simulink model "benchmark"  (Simulink 7.9) (continued)
== system benchmark/Wind Field == (continued)
      | image(imread('anemometer.jpg'))
      | 
      | port_label('output',1,'v_rot [m/s]')
      | port_label('output',2,'v_nac [m/s]')
      | 
      | 
      | port_label('input',1,'U_x [m/s]')
      | port_label('input',2,'WC [m]')
      | port_label('input',3,'a')
  Wind Speed6 : Subsystem  mask(Wind){}
      mask help: Calculates average over rotor and nacelle wind speeds based on wake effects
      mask init:
      | p=get_param(gcb,'UserData');
      mask Display:
      | image(imread('anemometer.jpg'))
      | 
      | port_label('output',1,'v_rot [m/s]')
      | port_label('output',2,'v_nac [m/s]')
      | 
      | 
      | port_label('input',1,'U_x [m/s]')
      | port_label('input',2,'WC [m]')
      | port_label('input',3,'a')
  Wind Speed7 : Subsystem  mask(Wind){}
      mask help: Calculates average over rotor and nacelle wind speeds based on wake effects
      mask init:
      | p=get_param(gcb,'UserData');
      mask Display:
      | image(imread('anemometer.jpg'))
      | 
      | port_label('output',1,'v_rot [m/s]')
      | port_label('output',2,'v_nac [m/s]')
      | 
      | 
      | port_label('input',1,'U_x [m/s]')
      | port_label('input',2,'WC [m]')
      | port_label('input',3,'a')
  Wind Speed8 : Subsystem  mask(Wind){}
      mask help: Calculates average over rotor and nacelle wind speeds based on wake effects
      mask init:
      | p=get_param(gcb,'UserData');
      mask Display:
      | image(imread('anemometer.jpg'))
      | 
      | port_label('output',1,'v_rot [m/s]')
      | port_label('output',2,'v_nac [m/s]')
      | 
      | 
      | port_label('input',1,'U_x [m/s]')
      | port_label('input',2,'WC [m]')
      | port_label('input',3,'a')
  Wind Speed9 : Subsystem  mask(Wind){}
      mask help: Calculates average over rotor and nacelle wind speeds based on wake effects
      mask init:
      | p=get_param(gcb,'UserData');
      mask Display:
      | image(imread('anemometer.jpg'))
      | 
      | port_label('output',1,'v_rot [m/s]')
      | port_label('output',2,'v_nac [m/s]')
      | 
      | 
      | port_label('input',1,'U_x [m/s]')
      | port_label('input',2,'WC [m]')
      | port_label('input',3,'a')
  aconcat : Concatenate  NumInputs=10  Mode=Multidimensional array  ConcatenateDimension=2
  rate1 : RateTransition  Deterministic=off  OutPortSampleTime=SWFTTs
  rate2 : RateTransition  Deterministic=off  OutPortSampleTime=SWFTTs
  wcconcat : Concatenate  NumInputs=10  Mode=Multidimensional array  ConcatenateDimension=2
  V_nac [m/s] : Outport
  V_rot [m/s] : Outport  Port=2
  wires:
  rate2/1 -> V_nac [m/s]/1
  rate1/1 -> V_rot [m/s]/1
  CTrate/1 -> CTdemux/1
  Vnacmux/1 -> rate2/1
  Vrotmux/1 -> rate1/1
  CT/1 -> CTrate/1
  CTdemux/1 -> Wake1/2(CT)
  Ambient Field/2(Uy) -> Wake1/1(uy), Wake2/1(uy), Wake3/1(uy), Wake4/1(uy), Wake5/1(uy), Wake6/1(uy), Wake7/1(uy), Wake8/1(uy), Wake9/1(uy), Wake10/1(uy)
  aconcat/1 -> Wind Speed1/3(a), Wind Speed2/3(a), Wind Speed3/3(a), Wind Speed4/3(a), Wind Speed5/3(a), Wind Speed6/3(a), Wind Speed7/3(a), Wind Speed8/3(a), Wind Speed9/3(a), Wind Speed10/3(a)  "wakeDeficit"
  wcconcat/1 -> Wind Speed1/2(w_c), Wind Speed2/2(w_c), Wind Speed3/2(w_c), Wind Speed4/2(w_c), Wind Speed5/2(w_c), Wind Speed6/2(w_c), Wind Speed7/2(w_c), Wind Speed8/2(w_c), Wind Speed9/2(w_c), Wind Speed10/2(w_c)  "wakeCenter"
  Ambient Field/1(Ux) -> Wind Speed1/1(ux), Wind Speed2/1(ux), Wind Speed3/1(ux), Wind Speed4/1(ux), Wind Speed5/1(ux), Wind Speed6/1(ux), Wind Speed7/1(ux), Wind Speed8/1(ux), Wind Speed9/1(ux), Wind Speed10/1(ux)
  Wake1/1(w_c) -> wcconcat/1
  Wake1/2(a) -> aconcat/1
  Wind Speed1/1(v_rot) -> Vrotmux/1
  Wind Speed1/2(v_nac) -> Vnacmux/1
  CTdemux/2 -> Wake2/2(CT)
  Wake2/1(w_c) -> wcconcat/2
  Wake2/2(a) -> aconcat/2
  Wind Speed2/1(v_rot) -> Vrotmux/2
  Wind Speed2/2(v_nac) -> Vnacmux/2
  CTdemux/3 -> Wake3/2(CT)
  Wake3/1(w_c) -> wcconcat/3
  Wake3/2(a) -> aconcat/3
  Wind Speed3/1(v_rot) -> Vrotmux/3
  Wind Speed3/2(v_nac) -> Vnacmux/3
  CTdemux/4 -> Wake4/2(CT)
  Wake4/1(w_c) -> wcconcat/4
  Wake4/2(a) -> aconcat/4
  Wind Speed4/1(v_rot) -> Vrotmux/4
  Wind Speed4/2(v_nac) -> Vnacmux/4
  CTdemux/5 -> Wake5/2(CT)
  Wake5/1(w_c) -> wcconcat/5
  Wake5/2(a) -> aconcat/5
  Wind Speed5/1(v_rot) -> Vrotmux/5
  Wind Speed5/2(v_nac) -> Vnacmux/5
  CTdemux/6 -> Wake6/2(CT)
  Wake6/1(w_c) -> wcconcat/6
  Wake6/2(a) -> aconcat/6
  Wind Speed6/1(v_rot) -> Vrotmux/6
  Wind Speed6/2(v_nac) -> Vnacmux/6
  CTdemux/7 -> Wake7/2(CT)
  Wake7/1(w_c) -> wcconcat/7
  Wake7/2(a) -> aconcat/7
  Wind Speed7/1(v_rot) -> Vrotmux/7
  Wind Speed7/2(v_nac) -> Vnacmux/7
  CTdemux/8 -> Wake8/2(CT)
  Wake8/1(w_c) -> wcconcat/8
  Wake8/2(a) -> aconcat/8
  Wind Speed8/1(v_rot) -> Vrotmux/8
  Wind Speed8/2(v_nac) -> Vnacmux/8
  CTdemux/9 -> Wake9/2(CT)
  Wake9/1(w_c) -> wcconcat/9
  Wake9/2(a) -> aconcat/9
  Wind Speed9/1(v_rot) -> Vrotmux/9
  Wind Speed9/2(v_nac) -> Vnacmux/9
  CTdemux/10 -> Wake10/2(CT)
  Wake10/1(w_c) -> wcconcat/10
  Wake10/2(a) -> aconcat/10
  Wind Speed10/1(v_rot) -> Vrotmux/10
  Wind Speed10/2(v_nac) -> Vnacmux/10

== system benchmark/Network Load/Power Load ==
  Demux : Demux  Tag=STV Demux  Outputs=1
  FromWs : FromWorkspace  Tag=STV FromWs  VariableName=tuvar  SampleTime=0  SigBuilderData=DataTag1  VnvData=DataTag2
      SigBuilderData=|
      | common.afterFinalStr = 'Extrapolate'
      | common.sampleTime = 0
      | dataSet.timeRange = [0 1000]
      | dataSet.name = 'Group 1'
      | sbobj.Groups.Name = 'Group 1'
      | sbobj.Groups.Signals.Name = 'Signal 1'
      | sbobj.Groups.Signals.XData = [0 25 100 200 500 1000]
      | sbobj.Groups.Signals.YData = [0.1 0.1 0.7 0.7 0.9 0.85]
  Signal 1 : Outport  Tag=STV Outport
  wires:
  FromWs/1 -> Demux/1
  Demux/1 -> Signal 1/1

== system benchmark/Post processing/Fatigue Computation ==

== system benchmark/Post processing/Wake Animation ==

== system benchmark/Post processing/Wind Field Animation ==

== system benchmark/Wind Field/Ambient Field ==
  Counter Free-Running : simulink/Sources/Counter Free-Running  NumBits=32  tsamp=wind.Ts
  Selector : Selector  NumberOfDimensions=2  InputPortWidth=3  IndexOptions=Select all,Index vector (port)  Indices=[1 3],1  OutputSizes=1,1
  Selector1 : Selector  NumberOfDimensions=2  InputPortWidth=3  IndexOptions=Select all,Index vector (port)  Indices=[1 3],1  OutputSizes=1,1
  Sum : Sum  Inputs=|++
  Turb x : Constant  Value=ceil(wind.grid.xsize/wind.grid.size)-wind.xgrids
  Ux_full : Constant  Value=wind.Ux
  Uy_full : Constant  Value=wind.Uy
  Ux : Outport
  Uy : Outport  Port=2
  wires:
  Ux_full/1 -> Selector/1
  Uy_full/1 -> Selector1/1
  Sum/1 -> Selector1/2, Selector/2
  Counter Free-Running/1 -> Sum/2
  Turb x/1 -> Sum/1
  Selector1/1 -> Uy/1
  Selector/1 -> Ux/1

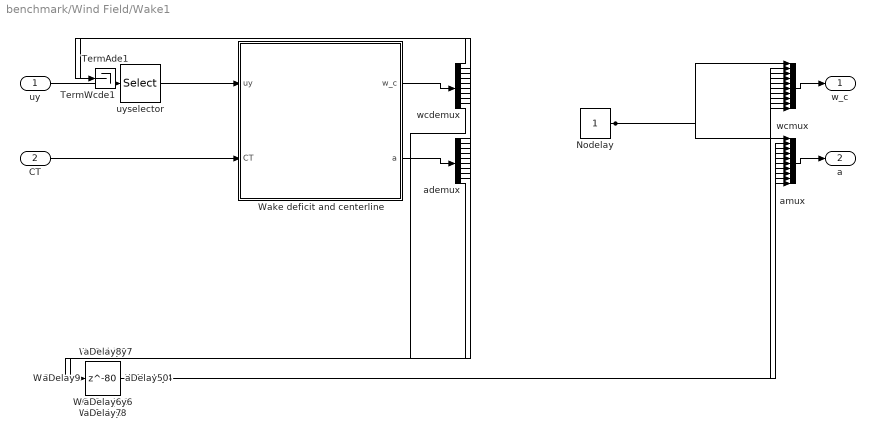
== system benchmark/Wind Field/Wake1 ==
  uy : Inport
  CT : Inport  Port=2
  Nodelay : Constant
  TermAde1 : Terminator
  TermWcde1 : Terminator
  WCDelay10 : Delay  DelayLength=120  InitialCondition=1000  InputProcessing=Inherited  SampleTime=1
  WCDelay2 : Delay  DelayLength=40  InitialCondition=1000  InputProcessing=Inherited  SampleTime=1
  WCDelay3 : Delay  DelayLength=80  InitialCondition=1000  InputProcessing=Inherited  SampleTime=1
  WCDelay4 : Delay  DelayLength=120  InitialCondition=1000  InputProcessing=Inherited  SampleTime=1
  WCDelay5 : Delay  DelayLength=20  InitialCondition=1000  InputProcessing=Inherited  SampleTime=1
  WCDelay6 : Delay  DelayLength=60  InitialCondition=1000  InputProcessing=Inherited  SampleTime=1
  WCDelay7 : Delay  DelayLength=100  InitialCondition=1000  InputProcessing=Inherited  SampleTime=1
  WCDelay8 : Delay  DelayLength=40  InitialCondition=1000  InputProcessing=Inherited  SampleTime=1
  WCDelay9 : Delay  DelayLength=80  InitialCondition=1000  InputProcessing=Inherited  SampleTime=1
  Wake deficit and centerline : MATLAB Function  TreatAsAtomicUnit=on
  aDelay10 : Delay  DelayLength=120  InitialCondition=1  InputProcessing=Inherited  SampleTime=1
  aDelay2 : Delay  DelayLength=40  InitialCondition=1  InputProcessing=Inherited  SampleTime=1
  aDelay3 : Delay  DelayLength=80  InitialCondition=1  InputProcessing=Inherited  SampleTime=1
  aDelay4 : Delay  DelayLength=120  InitialCondition=1  InputProcessing=Inherited  SampleTime=1
  aDelay5 : Delay  DelayLength=20  InitialCondition=1  InputProcessing=Inherited  SampleTime=1
  aDelay6 : Delay  DelayLength=60  InitialCondition=1  InputProcessing=Inherited  SampleTime=1
  aDelay7 : Delay  DelayLength=100  InitialCondition=1  InputProcessing=Inherited  SampleTime=1
  aDelay8 : Delay  DelayLength=40  InitialCondition=1  InputProcessing=Inherited  SampleTime=1
  aDelay9 : Delay  DelayLength=80  InitialCondition=1  InputProcessing=Inherited  SampleTime=1
  ademux : Demux  Outputs=10
  amux : Mux  Inputs=10
  uyselector : Selector  NumberOfDimensions=2  InputPortWidth=3  IndexOptions=Select all,Index vector (dialog)  Indices=[1 3],p.wt.num  OutputSizes=1,1
  wcdemux : Demux  Outputs=10
  wcmux : Mux  Inputs=10
  w_c : Outport
  a : Outport  Port=2
  wires:
  amux/1 -> a/1
  wcmux/1 -> w_c/1
  Wake deficit and centerline/2(a) -> ademux/1
  Wake deficit and centerline/1(w_c) -> wcdemux/1
  uyselector/1 -> Wake deficit and centerline/1(uy)
  uy/1 -> uyselector/1
  CT/1 -> Wake deficit and centerline/2(CT)
  ademux/1 -> TermAde1/1
  wcdemux/1 -> TermWcde1/1
  Nodelay/1 -> amux/1, wcmux/1
  ademux/2 -> aDelay2/1
  aDelay2/1 -> amux/2
  wcdemux/2 -> WCDelay2/1
  WCDelay2/1 -> wcmux/2
  ademux/3 -> aDelay3/1
  aDelay3/1 -> amux/3
  wcdemux/3 -> WCDelay3/1
  WCDelay3/1 -> wcmux/3
  ademux/4 -> aDelay4/1
  aDelay4/1 -> amux/4
  wcdemux/4 -> WCDelay4/1
  WCDelay4/1 -> wcmux/4
  ademux/5 -> aDelay5/1
  aDelay5/1 -> amux/5
  wcdemux/5 -> WCDelay5/1
  WCDelay5/1 -> wcmux/5
  ademux/6 -> aDelay6/1
  aDelay6/1 -> amux/6
  wcdemux/6 -> WCDelay6/1
  WCDelay6/1 -> wcmux/6
  ademux/7 -> aDelay7/1
  aDelay7/1 -> amux/7
  wcdemux/7 -> WCDelay7/1
  WCDelay7/1 -> wcmux/7
  ademux/8 -> aDelay8/1
  aDelay8/1 -> amux/8
  wcdemux/8 -> WCDelay8/1
  WCDelay8/1 -> wcmux/8
  ademux/9 -> aDelay9/1
  aDelay9/1 -> amux/9
  wcdemux/9 -> WCDelay9/1
  WCDelay9/1 -> wcmux/9
  ademux/10 -> aDelay10/1
  aDelay10/1 -> amux/10
  wcdemux/10 -> WCDelay10/1
  WCDelay10/1 -> wcmux/10

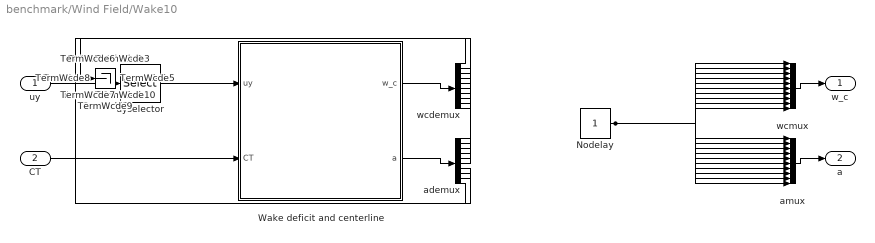
== system benchmark/Wind Field/Wake10 ==
  uy : Inport
  CT : Inport  Port=2
  Nodelay : Constant
  TermAde{1..10} (10 alike) : Terminator
  TermWcde{1..10} (10 alike) : Terminator
  Wake deficit and centerline : MATLAB Function  TreatAsAtomicUnit=on
  ademux : Demux  Outputs=10
  amux : Mux  Inputs=10
  uyselector : Selector  NumberOfDimensions=2  InputPortWidth=3  IndexOptions=Select all,Index vector (dialog)  Indices=[1 3],p.wt.num  OutputSizes=1,1
  wcdemux : Demux  Outputs=10
  wcmux : Mux  Inputs=10
  w_c : Outport
  a : Outport  Port=2
  wires:
  amux/1 -> a/1
  wcmux/1 -> w_c/1
  Wake deficit and centerline/2(a) -> ademux/1
  Wake deficit and centerline/1(w_c) -> wcdemux/1
  uyselector/1 -> Wake deficit and centerline/1(uy)
  uy/1 -> uyselector/1
  CT/1 -> Wake deficit and centerline/2(CT)
  ademux/1 -> TermAde1/1
  wcdemux/1 -> TermWcde1/1
  Nodelay/1 -> amux/1, wcmux/1, amux/2, wcmux/2, amux/3, wcmux/3, amux/4, wcmux/4, amux/5, wcmux/5, amux/6, wcmux/6, amux/7, wcmux/7, amux/8, wcmux/8, amux/9, wcmux/9, amux/10, wcmux/10
  ademux/2 -> TermAde2/1
  wcdemux/2 -> TermWcde2/1
  ademux/3 -> TermAde3/1
  wcdemux/3 -> TermWcde3/1
  ademux/4 -> TermAde4/1
  wcdemux/4 -> TermWcde4/1
  ademux/5 -> TermAde5/1
  wcdemux/5 -> TermWcde5/1
  ademux/6 -> TermAde6/1
  wcdemux/6 -> TermWcde6/1
  ademux/7 -> TermAde7/1
  wcdemux/7 -> TermWcde7/1
  ademux/8 -> TermAde8/1
  wcdemux/8 -> TermWcde8/1
  ademux/9 -> TermAde9/1
  wcdemux/9 -> TermWcde9/1
  ademux/10 -> TermAde10/1
  wcdemux/10 -> TermWcde10/1

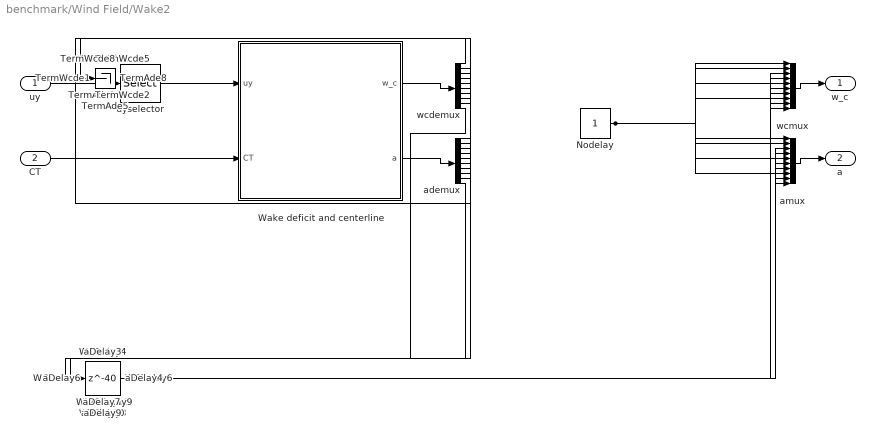
== system benchmark/Wind Field/Wake2 ==
  uy : Inport
  CT : Inport  Port=2
  Nodelay : Constant
  TermAde{1..2,5,8} (4 alike) : Terminator
  TermWcde{1..2,5,8} (4 alike) : Terminator
  WCDelay10 : Delay  DelayLength=80  InitialCondition=1000  InputProcessing=Inherited  SampleTime=1
  WCDelay3 : Delay  DelayLength=40  InitialCondition=1000  InputProcessing=Inherited  SampleTime=1
  WCDelay4 : Delay  DelayLength=80  InitialCondition=1000  InputProcessing=Inherited  SampleTime=1
  WCDelay6 : Delay  DelayLength=20  InitialCondition=1000  InputProcessing=Inherited  SampleTime=1
  WCDelay7 : Delay  DelayLength=60  InitialCondition=1000  InputProcessing=Inherited  SampleTime=1
  WCDelay9 : Delay  DelayLength=40  InitialCondition=1000  InputProcessing=Inherited  SampleTime=1
  Wake deficit and centerline : MATLAB Function  TreatAsAtomicUnit=on
  aDelay10 : Delay  DelayLength=80  InitialCondition=1  InputProcessing=Inherited  SampleTime=1
  aDelay3 : Delay  DelayLength=40  InitialCondition=1  InputProcessing=Inherited  SampleTime=1
  aDelay4 : Delay  DelayLength=80  InitialCondition=1  InputProcessing=Inherited  SampleTime=1
  aDelay6 : Delay  DelayLength=20  InitialCondition=1  InputProcessing=Inherited  SampleTime=1
  aDelay7 : Delay  DelayLength=60  InitialCondition=1  InputProcessing=Inherited  SampleTime=1
  aDelay9 : Delay  DelayLength=40  InitialCondition=1  InputProcessing=Inherited  SampleTime=1
  ademux : Demux  Outputs=10
  amux : Mux  Inputs=10
  uyselector : Selector  NumberOfDimensions=2  InputPortWidth=3  IndexOptions=Select all,Index vector (dialog)  Indices=[1 3],p.wt.num  OutputSizes=1,1
  wcdemux : Demux  Outputs=10
  wcmux : Mux  Inputs=10
  w_c : Outport
  a : Outport  Port=2
  wires:
  amux/1 -> a/1
  wcmux/1 -> w_c/1
  Wake deficit and centerline/2(a) -> ademux/1
  Wake deficit and centerline/1(w_c) -> wcdemux/1
  uyselector/1 -> Wake deficit and centerline/1(uy)
  uy/1 -> uyselector/1
  CT/1 -> Wake deficit and centerline/2(CT)
  ademux/1 -> TermAde1/1
  wcdemux/1 -> TermWcde1/1
  Nodelay/1 -> amux/1, wcmux/1, amux/2, wcmux/2, amux/5, wcmux/5, amux/8, wcmux/8
  ademux/2 -> TermAde2/1
  wcdemux/2 -> TermWcde2/1
  ademux/3 -> aDelay3/1
  aDelay3/1 -> amux/3
  wcdemux/3 -> WCDelay3/1
  WCDelay3/1 -> wcmux/3
  ademux/4 -> aDelay4/1
  aDelay4/1 -> amux/4
  wcdemux/4 -> WCDelay4/1
  WCDelay4/1 -> wcmux/4
  ademux/5 -> TermAde5/1
  wcdemux/5 -> TermWcde5/1
  ademux/6 -> aDelay6/1
  aDelay6/1 -> amux/6
  wcdemux/6 -> WCDelay6/1
  WCDelay6/1 -> wcmux/6
  ademux/7 -> aDelay7/1
  aDelay7/1 -> amux/7
  wcdemux/7 -> WCDelay7/1
  WCDelay7/1 -> wcmux/7
  ademux/8 -> TermAde8/1
  wcdemux/8 -> TermWcde8/1
  ademux/9 -> aDelay9/1
  aDelay9/1 -> amux/9
  wcdemux/9 -> WCDelay9/1
  WCDelay9/1 -> wcmux/9
  ademux/10 -> aDelay10/1
  aDelay10/1 -> amux/10
  wcdemux/10 -> WCDelay10/1
  WCDelay10/1 -> wcmux/10

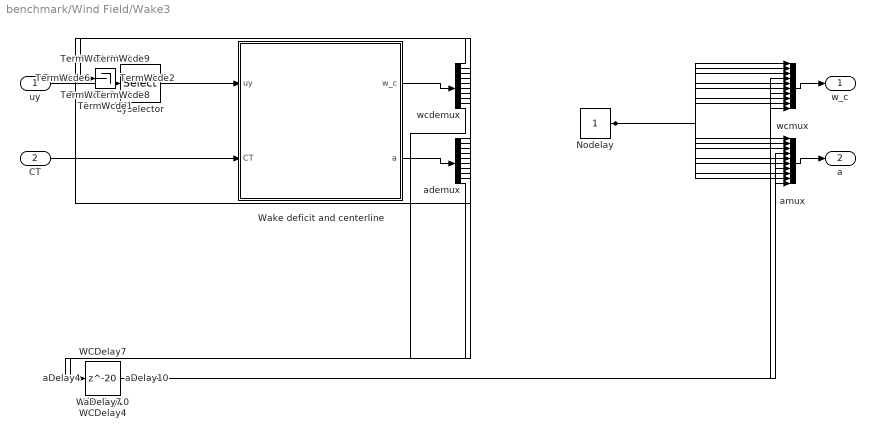
== system benchmark/Wind Field/Wake3 ==
  uy : Inport
  CT : Inport  Port=2
  Nodelay : Constant
  TermAde{1..3,5..6,8..9} (7 alike) : Terminator
  TermWcde{1..3,5..6,8..9} (7 alike) : Terminator
  WCDelay10 : Delay  DelayLength=40  InitialCondition=1000  InputProcessing=Inherited  SampleTime=1
  WCDelay4 : Delay  DelayLength=40  InitialCondition=1000  InputProcessing=Inherited  SampleTime=1
  WCDelay7 : Delay  DelayLength=20  InitialCondition=1000  InputProcessing=Inherited  SampleTime=1
  Wake deficit and centerline : MATLAB Function  TreatAsAtomicUnit=on
  aDelay10 : Delay  DelayLength=40  InitialCondition=1  InputProcessing=Inherited  SampleTime=1
  aDelay4 : Delay  DelayLength=40  InitialCondition=1  InputProcessing=Inherited  SampleTime=1
  aDelay7 : Delay  DelayLength=20  InitialCondition=1  InputProcessing=Inherited  SampleTime=1
  ademux : Demux  Outputs=10
  amux : Mux  Inputs=10
  uyselector : Selector  NumberOfDimensions=2  InputPortWidth=3  IndexOptions=Select all,Index vector (dialog)  Indices=[1 3],p.wt.num  OutputSizes=1,1
  wcdemux : Demux  Outputs=10
  wcmux : Mux  Inputs=10
  w_c : Outport
  a : Outport  Port=2
  wires:
  amux/1 -> a/1
  wcmux/1 -> w_c/1
  Wake deficit and centerline/2(a) -> ademux/1
  Wake deficit and centerline/1(w_c) -> wcdemux/1
  uyselector/1 -> Wake deficit and centerline/1(uy)
  uy/1 -> uyselector/1
  CT/1 -> Wake deficit and centerline/2(CT)
  ademux/1 -> TermAde1/1
  wcdemux/1 -> TermWcde1/1
  Nodelay/1 -> amux/1, wcmux/1, amux/2, wcmux/2, amux/3, wcmux/3, amux/5, wcmux/5, amux/6, wcmux/6, amux/8, wcmux/8, amux/9, wcmux/9
  ademux/2 -> TermAde2/1
  wcdemux/2 -> TermWcde2/1
  ademux/3 -> TermAde3/1
  wcdemux/3 -> TermWcde3/1
  ademux/4 -> aDelay4/1
  aDelay4/1 -> amux/4
  wcdemux/4 -> WCDelay4/1
  WCDelay4/1 -> wcmux/4
  ademux/5 -> TermAde5/1
  wcdemux/5 -> TermWcde5/1
  ademux/6 -> TermAde6/1
  wcdemux/6 -> TermWcde6/1
  ademux/7 -> aDelay7/1
  aDelay7/1 -> amux/7
  wcdemux/7 -> WCDelay7/1
  WCDelay7/1 -> wcmux/7
  ademux/8 -> TermAde8/1
  wcdemux/8 -> TermWcde8/1
  ademux/9 -> TermAde9/1
  wcdemux/9 -> TermWcde9/1
  ademux/10 -> aDelay10/1
  aDelay10/1 -> amux/10
  wcdemux/10 -> WCDelay10/1
  WCDelay10/1 -> wcmux/10

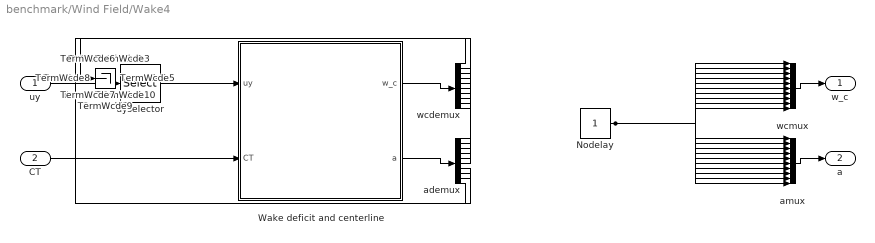
== system benchmark/Wind Field/Wake4 ==
  uy : Inport
  CT : Inport  Port=2
  Nodelay : Constant
  TermAde{1..10} (10 alike) : Terminator
  TermWcde{1..10} (10 alike) : Terminator
  Wake deficit and centerline : MATLAB Function  TreatAsAtomicUnit=on
  ademux : Demux  Outputs=10
  amux : Mux  Inputs=10
  uyselector : Selector  NumberOfDimensions=2  InputPortWidth=3  IndexOptions=Select all,Index vector (dialog)  Indices=[1 3],p.wt.num  OutputSizes=1,1
  wcdemux : Demux  Outputs=10
  wcmux : Mux  Inputs=10
  w_c : Outport
  a : Outport  Port=2
  wires:
  amux/1 -> a/1
  wcmux/1 -> w_c/1
  Wake deficit and centerline/2(a) -> ademux/1
  Wake deficit and centerline/1(w_c) -> wcdemux/1
  uyselector/1 -> Wake deficit and centerline/1(uy)
  uy/1 -> uyselector/1
  CT/1 -> Wake deficit and centerline/2(CT)
  ademux/1 -> TermAde1/1
  wcdemux/1 -> TermWcde1/1
  Nodelay/1 -> amux/1, wcmux/1, amux/2, wcmux/2, amux/3, wcmux/3, amux/4, wcmux/4, amux/5, wcmux/5, amux/6, wcmux/6, amux/7, wcmux/7, amux/8, wcmux/8, amux/9, wcmux/9, amux/10, wcmux/10
  ademux/2 -> TermAde2/1
  wcdemux/2 -> TermWcde2/1
  ademux/3 -> TermAde3/1
  wcdemux/3 -> TermWcde3/1
  ademux/4 -> TermAde4/1
  wcdemux/4 -> TermWcde4/1
  ademux/5 -> TermAde5/1
  wcdemux/5 -> TermWcde5/1
  ademux/6 -> TermAde6/1
  wcdemux/6 -> TermWcde6/1
  ademux/7 -> TermAde7/1
  wcdemux/7 -> TermWcde7/1
  ademux/8 -> TermAde8/1
  wcdemux/8 -> TermWcde8/1
  ademux/9 -> TermAde9/1
  wcdemux/9 -> TermWcde9/1
  ademux/10 -> TermAde10/1
  wcdemux/10 -> TermWcde10/1

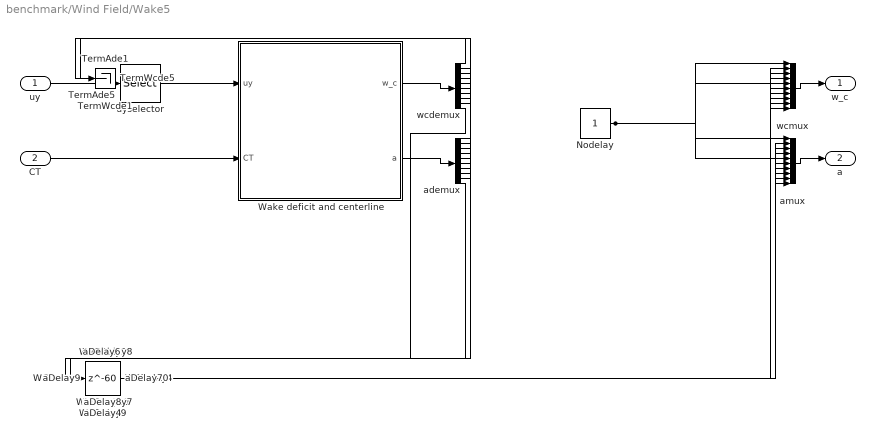
== system benchmark/Wind Field/Wake5 ==
  uy : Inport
  CT : Inport  Port=2
  Nodelay : Constant
  TermAde1 : Terminator
  TermAde5 : Terminator
  TermWcde1 : Terminator
  TermWcde5 : Terminator
  WCDelay10 : Delay  DelayLength=100  InitialCondition=1500  InputProcessing=Inherited  SampleTime=1
  WCDelay2 : Delay  DelayLength=20  InitialCondition=1500  InputProcessing=Inherited  SampleTime=1
  WCDelay3 : Delay  DelayLength=60  InitialCondition=1500  InputProcessing=Inherited  SampleTime=1
  WCDelay4 : Delay  DelayLength=100  InitialCondition=1500  InputProcessing=Inherited  SampleTime=1
  WCDelay6 : Delay  DelayLength=40  InitialCondition=1500  InputProcessing=Inherited  SampleTime=1
  WCDelay7 : Delay  DelayLength=80  InitialCondition=1500  InputProcessing=Inherited  SampleTime=1
  WCDelay8 : Delay  DelayLength=20  InitialCondition=1500  InputProcessing=Inherited  SampleTime=1
  WCDelay9 : Delay  DelayLength=60  InitialCondition=1500  InputProcessing=Inherited  SampleTime=1
  Wake deficit and centerline : MATLAB Function  TreatAsAtomicUnit=on
  aDelay10 : Delay  DelayLength=100  InitialCondition=1  InputProcessing=Inherited  SampleTime=1
  aDelay2 : Delay  DelayLength=20  InitialCondition=1  InputProcessing=Inherited  SampleTime=1
  aDelay3 : Delay  DelayLength=60  InitialCondition=1  InputProcessing=Inherited  SampleTime=1
  aDelay4 : Delay  DelayLength=100  InitialCondition=1  InputProcessing=Inherited  SampleTime=1
  aDelay6 : Delay  DelayLength=40  InitialCondition=1  InputProcessing=Inherited  SampleTime=1
  aDelay7 : Delay  DelayLength=80  InitialCondition=1  InputProcessing=Inherited  SampleTime=1
  aDelay8 : Delay  DelayLength=20  InitialCondition=1  InputProcessing=Inherited  SampleTime=1
  aDelay9 : Delay  DelayLength=60  InitialCondition=1  InputProcessing=Inherited  SampleTime=1
  ademux : Demux  Outputs=10
  amux : Mux  Inputs=10
  uyselector : Selector  NumberOfDimensions=2  InputPortWidth=3  IndexOptions=Select all,Index vector (dialog)  Indices=[1 3],p.wt.num  OutputSizes=1,1
  wcdemux : Demux  Outputs=10
  wcmux : Mux  Inputs=10
  w_c : Outport
  a : Outport  Port=2
  wires:
  amux/1 -> a/1
  wcmux/1 -> w_c/1
  Wake deficit and centerline/2(a) -> ademux/1
  Wake deficit and centerline/1(w_c) -> wcdemux/1
  uyselector/1 -> Wake deficit and centerline/1(uy)
  uy/1 -> uyselector/1
  CT/1 -> Wake deficit and centerline/2(CT)
  ademux/1 -> TermAde1/1
  wcdemux/1 -> TermWcde1/1
  Nodelay/1 -> amux/1, wcmux/1, amux/5, wcmux/5
  ademux/2 -> aDelay2/1
  aDelay2/1 -> amux/2
  wcdemux/2 -> WCDelay2/1
  WCDelay2/1 -> wcmux/2
  ademux/3 -> aDelay3/1
  aDelay3/1 -> amux/3
  wcdemux/3 -> WCDelay3/1
  WCDelay3/1 -> wcmux/3
  ademux/4 -> aDelay4/1
  aDelay4/1 -> amux/4
  wcdemux/4 -> WCDelay4/1
  WCDelay4/1 -> wcmux/4
  ademux/5 -> TermAde5/1
  wcdemux/5 -> TermWcde5/1
  ademux/6 -> aDelay6/1
  aDelay6/1 -> amux/6
  wcdemux/6 -> WCDelay6/1
  WCDelay6/1 -> wcmux/6
  ademux/7 -> aDelay7/1
  aDelay7/1 -> amux/7
  wcdemux/7 -> WCDelay7/1
  WCDelay7/1 -> wcmux/7
  ademux/8 -> aDelay8/1
  aDelay8/1 -> amux/8
  wcdemux/8 -> WCDelay8/1
  WCDelay8/1 -> wcmux/8
  ademux/9 -> aDelay9/1
  aDelay9/1 -> amux/9
  wcdemux/9 -> WCDelay9/1
  WCDelay9/1 -> wcmux/9
  ademux/10 -> aDelay10/1
  aDelay10/1 -> amux/10
  wcdemux/10 -> WCDelay10/1
  WCDelay10/1 -> wcmux/10

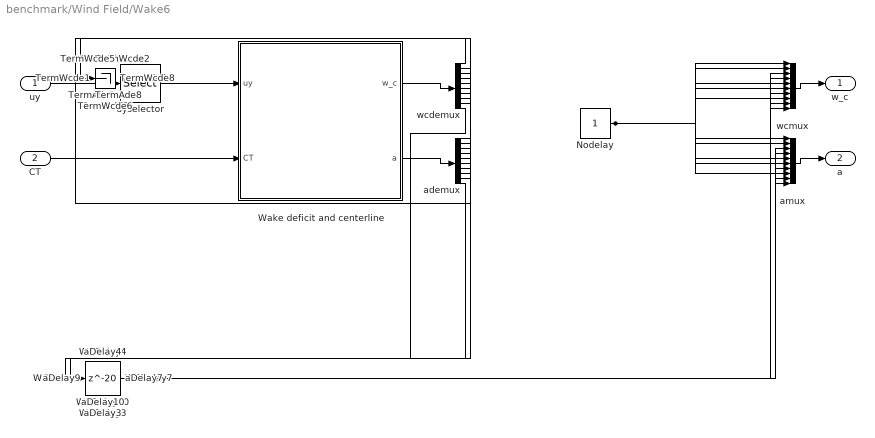
== system benchmark/Wind Field/Wake6 ==
  uy : Inport
  CT : Inport  Port=2
  Nodelay : Constant
  TermAde{1..2,5..6,8} (5 alike) : Terminator
  TermWcde{1..2,5..6,8} (5 alike) : Terminator
  WCDelay10 : Delay  DelayLength=60  InitialCondition=1500  InputProcessing=Inherited  SampleTime=1
  WCDelay3 : Delay  DelayLength=20  InitialCondition=1500  InputProcessing=Inherited  SampleTime=1
  WCDelay4 : Delay  DelayLength=60  InitialCondition=1500  InputProcessing=Inherited  SampleTime=1
  WCDelay7 : Delay  DelayLength=40  InitialCondition=1500  InputProcessing=Inherited  SampleTime=1
  WCDelay9 : Delay  DelayLength=20  InitialCondition=1500  InputProcessing=Inherited  SampleTime=1
  Wake deficit and centerline : MATLAB Function  TreatAsAtomicUnit=on
  aDelay10 : Delay  DelayLength=60  InitialCondition=1  InputProcessing=Inherited  SampleTime=1
  aDelay3 : Delay  DelayLength=20  InitialCondition=1  InputProcessing=Inherited  SampleTime=1
  aDelay4 : Delay  DelayLength=60  InitialCondition=1  InputProcessing=Inherited  SampleTime=1
  aDelay7 : Delay  DelayLength=40  InitialCondition=1  InputProcessing=Inherited  SampleTime=1
  aDelay9 : Delay  DelayLength=20  InitialCondition=1  InputProcessing=Inherited  SampleTime=1
  ademux : Demux  Outputs=10
  amux : Mux  Inputs=10
  uyselector : Selector  NumberOfDimensions=2  InputPortWidth=3  IndexOptions=Select all,Index vector (dialog)  Indices=[1 3],p.wt.num  OutputSizes=1,1
  wcdemux : Demux  Outputs=10
  wcmux : Mux  Inputs=10
  w_c : Outport
  a : Outport  Port=2
  wires:
  amux/1 -> a/1
  wcmux/1 -> w_c/1
  Wake deficit and centerline/2(a) -> ademux/1
  Wake deficit and centerline/1(w_c) -> wcdemux/1
  uyselector/1 -> Wake deficit and centerline/1(uy)
  uy/1 -> uyselector/1
  CT/1 -> Wake deficit and centerline/2(CT)
  ademux/1 -> TermAde1/1
  wcdemux/1 -> TermWcde1/1
  Nodelay/1 -> amux/1, wcmux/1, amux/2, wcmux/2, amux/5, wcmux/5, amux/6, wcmux/6, amux/8, wcmux/8
  ademux/2 -> TermAde2/1
  wcdemux/2 -> TermWcde2/1
  ademux/3 -> aDelay3/1
  aDelay3/1 -> amux/3
  wcdemux/3 -> WCDelay3/1
  WCDelay3/1 -> wcmux/3
  ademux/4 -> aDelay4/1
  aDelay4/1 -> amux/4
  wcdemux/4 -> WCDelay4/1
  WCDelay4/1 -> wcmux/4
  ademux/5 -> TermAde5/1
  wcdemux/5 -> TermWcde5/1
  ademux/6 -> TermAde6/1
  wcdemux/6 -> TermWcde6/1
  ademux/7 -> aDelay7/1
  aDelay7/1 -> amux/7
  wcdemux/7 -> WCDelay7/1
  WCDelay7/1 -> wcmux/7
  ademux/8 -> TermAde8/1
  wcdemux/8 -> TermWcde8/1
  ademux/9 -> aDelay9/1
  aDelay9/1 -> amux/9
  wcdemux/9 -> WCDelay9/1
  WCDelay9/1 -> wcmux/9
  ademux/10 -> aDelay10/1
  aDelay10/1 -> amux/10
  wcdemux/10 -> WCDelay10/1
  WCDelay10/1 -> wcmux/10
